simulink model "MCVT_Integration"  (R2020a)
settings: Solver=ode8  StartTime=0.0  StopTime=T_end  FixedStep=dt  RelTol=1e-3  EnableMultiTasking=on
values from neighbouring files (symbols this model uses):
  assumed_power_voltage = 1.25e2    (= 125)    [MCVT_Input_File.m]    (also in MCVT_Run_QuickCheck.m)
  battery_power_toggle = 1    [MCVT_Input_File.m]    (also in MCVT_Run_QuickCheck.m)
  battery_trickle_charge_param = 0.1    [MCVT_Input_File.m]    (also in MCVT_Run_QuickCheck.m)
  battery_trickle_leakage_param = 0.01    [MCVT_Input_File.m]    (also in MCVT_Run_QuickCheck.m)
  damage_case_id = 3    [MCVT_Input_File.m]    (also in MCVT_Run_QuickCheck.m = 1)
  dt = 1/fs    (= 0.001)    [MCVT_Run_QuickCheck.m]    (also in MCVT_Sim_Set.m)
  dt_2 = 1/fs_2    (= 0.001)    [MCVT_Run_QuickCheck.m]    (also in MCVT_Sim_Set.m)
  dt_3 = 1/fs_3    (= 0.001)    [MCVT_Run_QuickCheck.m]    (also in MCVT_Sim_Set.m)
  dt_5 = 1/fs_5    (= 0.005)    [MCVT_Run_QuickCheck.m]    (also in MCVT_Sim_Set.m)
  dt_8 = 1/fs_8    (= 0.05)    [MCVT_Run_QuickCheck.m]    (also in MCVT_Sim_Set.m)
  dt_9 = 1/fs_9    (= 0.001)    [MCVT_Run_QuickCheck.m]    (also in MCVT_Sim_Set.m)
  dust_met_duration = 3    [MCVT_Input_File.m]    (also in MCVT_Run_QuickCheck.m = 2)
  ECLSS_indoor_set_temperature = 298.15    [MCVT_Input_File.m]    (also in MCVT_Run_QuickCheck.m)
  energy_store_max_o = 150    [MCVT_Input_File.m]    (also in MCVT_Run_QuickCheck.m)
  energy_store_o = 120    [MCVT_Input_File.m]    (also in MCVT_Run_QuickCheck.m)
  fs = 1000    [MCVT_Run_QuickCheck.m]    (also in MCVT_Sim_Set.m)
  gain_charge_param = 1    [MCVT_Input_File.m]    (also in MCVT_Run_QuickCheck.m)
  ground_temperature = 373    [MCVT_Habitat_Design_Input_File.m]    (also in MCVT_Run_QuickCheck.m)
  I_max_charge = 800    [MCVT_Input_File.m]    (also in MCVT_Run_QuickCheck.m)
  I_max_discharge = 800    [MCVT_Input_File.m]    (also in MCVT_Run_QuickCheck.m)
  initial_pressure = 1e5    (= 100000)    [MCVT_Input_File.m]    (also in MCVT_Run_QuickCheck.m)
  initial_temperature = 295    [MCVT_Input_File.m]    (also in MCVT_Run_QuickCheck.m)
  interior_leak_rate = 0.00415    [MCVT_Input_File.m]    (also in MCVT_Run_QuickCheck.m)
  launch_dust_accum_start_time = 10    [MCVT_Input_File.m]    (also in MCVT_Run_QuickCheck.m = 2)
  launch_dust_accum_stop_time = 12    [MCVT_Input_File.m]    (also in MCVT_Run_QuickCheck.m = 3)
  meteorite_impact_time = 2    [MCVT_Input_File.m]    (also in MCVT_Run_QuickCheck.m = 1)
  nuclear_power_toggle = 1    [MCVT_Input_File.m]    (also in MCVT_Run_QuickCheck.m)
  print_interval = 30    [MCVT_Input_File.m]
  SOC_lower_thres = 0.5    [MCVT_Input_File.m]    (also in MCVT_Run_QuickCheck.m)
  SOC_upper_thres = 0.9    [MCVT_Input_File.m]    (also in MCVT_Run_QuickCheck.m)
  solar_power_toggle = 1    [MCVT_Input_File.m]    (also in MCVT_Run_QuickCheck.m)
  space_temperature = 4    [MCVT_Habitat_Design_Input_File.m]    (also in MCVT_Run_QuickCheck.m)
  Start_angle = 90    [MCVT_Input_File.m]    (also in MCVT_Run_QuickCheck.m)
undefined symbols (not defined in the file or in its folder): A_flow_restrictor, ACTIVITY_LOOKUP_TABLE, air, Aref, Bmap, Bmap2, Bmap3, comms, DiscIntNeverNeededParam, DmgSPL, dust_parameters, ECLSS_dust_damage_repair_time, ECLSS_heater_capacity, ECLSS_IPS_restrictor, ECLSS_IPS_setpointPressure, ECLSS_paint_damage_repair_time, ECLSS_radiatorLoop_discreteNumber, ECLSS_Vst, emptyActivity, expected_heat_add, FI, habitat, HIEM2SMM, HIEM2STM, HIEMDamageElements, impact_numbering, initial_SPLT_damage_index, input_air, input_Cond, input_CP, input_discretization, input_EV, input_Evap, input_radiatorLoop, K_ECLSS_dust_single, K_ECLSS_paint_single, K_Structure_damage_single, Kref, kW_to_W, meteoroidDustDep, mFRF, ndelays, nominalDustDep, noverlap, nuclear_toggle_gain, output_limits, panel_paint0, Ps0, reference_temperature, RepairTime_ECLSS_factor, Sensor_Location_SMM, SMM_T0, SMM_T1, SMM_T2, SMM_T3, SMM_T4, SMM_T4_0, SMM_T4_1, SMM_T4_2, solar_FDD_K ... (+73)
libraries: neural x350, simulink x39, xpcutilitieslib x7, xpcrtudplib x7, dspstat3 x1
(part 29 of 43)

== system MCVT_Integration/Sys2 - Structural System/Sys2_Structural_System/Fault Detection and Diagnosis (FDD) with Binary Classifer/Pattern Recognition Neural Network/Segment 4/Layer 2/Delays 1 ==
  "p{2}" : Inport  PortDimensions=20
      mask Display: e = 0.06;c1 = [120 230 180]/255;c2 = ([120 230 180]+0)/(256+128);c3 = ([120 230 180]+128)/(256+128);patch([0 1 1 0],[0 0 1 1],c1);patch([0 1 1 1-e 1-e 0+e],[0 0 1 1-e 0+e 0+e],c2);patch([0 0 1 1-e 0+e 0+e],[0 1 1 1-e 1-e 0+e],c3);plot([0 1 1 0 0],[0 0 1 1 0]);
  mux : Mux  Inputs=1
      mask Display: e = 0.06;c1 = [120 150 230]/255;c2 = ([120 150 230]+0)/383;c3 = ([120 150 230]+128)/383;patch([0 1 1 0],[0 0 1 1],c1);patch([0 1 1 1-e 1-e 0+e],[0 0 1 1-e 0+e 0+e],c2);patch([0 0 1 1-e 0+e 0+e],[0 1 1 1-e 1-e 0+e],c3);plot([0 1 1 0 0],[0 0 1 1 0]);disp('Mux')
  "pd{2,1}" : Outport
      mask Display: e = 0.06;c1 = [120 230 180]/255;c2 = ([120 230 180]+0)/(256+128);c3 = ([120 230 180]+128)/(256+128);patch([0 1 1 0],[0 0 1 1],c1);patch([0 1 1 1-e 1-e 0+e],[0 0 1 1-e 0+e 0+e],c2);patch([0 0 1 1-e 0+e 0+e],[0 1 1 1-e 1-e 0+e],c3);plot([0 1 1 0 0],[0 0 1 1 0]);
  wires:
  "p{2}"/1 -> mux/1
  mux/1 -> "pd{2,1}"/1

== system MCVT_Integration/Sys2 - Structural System/Sys2_Structural_System/Fault Detection and Diagnosis (FDD) with Binary Classifer/Pattern Recognition Neural Network/Segment 4/Layer 2/LW{2,1} ==
  "ad{2,1}" : Inport  PortDimensions=20
      mask Display: e = 0.06;c1 = [120 230 180]/255;c2 = ([120 230 180]+0)/(256+128);c3 = ([120 230 180]+128)/(256+128);patch([0 1 1 0],[0 0 1 1],c1);patch([0 1 1 1-e 1-e 0+e],[0 0 1 1-e 0+e 0+e],c2);patch([0 0 1 1-e 0+e 0+e],[0 1 1 1-e 1-e 0+e],c3);plot([0 1 1 0 0],[0 0 1 1 0]);
  "IW{2,1}(1,:)'" : Constant  Value=[0.306057142007762739144283159475889988243579864501953125;0.310512128739632020657523980844416655600070953369140625;-0.29480112676645597868940740227117203176021575927734375;0.2026576403739423770655747603086638264358043670654296875;0.0186213755057179040919290713418376981280744075775146484375;-0.62303529414836733568705540164955891668796539306640625;0.27996475650757235431598246577777899801731109619140625;-0.469892300787330519096229863862390629947185516357421875;0.349973395308618318022553239643457345664501190185546875;-0.476340885668002533837039891295717097818851470947265625;-0.02019900162674355403868986513771233148872852325439453125;0.472241782546273636267386564213666133582592010498046875;-0.07055543952916958072574260540932300500571727752685546875;-0.678306634812040787352316328906454145908355712890625;0.296746899937958996673614819883368909358978271484375;0.198279570842823271714649990826728753745555877685546875;-0.025708135562139890206712067310945712961256504058837890625;-0.338335032564995363824067453606403432786464691162109375;0.08612698156654709225055199794951477088034152984619140625;-0.68933092867270528980583321754238568246364593505859375]
      mask Display: e = 0.06;c1 = [120 150 230]/255;c2 = ([120 150 230]+0)/383;c3 = ([120 150 230]+128)/383;patch([0 1 1 0],[0 0 1 1],c1);patch([0 1 1 1-e 1-e 0+e],[0 0 1 1-e 0+e 0+e],c2);patch([0 0 1 1-e 0+e 0+e],[0 1 1 1-e 1-e 0+e],c3);plot([0 1 1 0 0],[0 0 1 1 0]);disp('weights');
  "IW{2,1}(10,:)'" : Constant  Value=[-0.454412246804836328362142694459180347621440887451171875;-0.4824565986072466561296323561691679060459136962890625;-0.275395655377960679732751714254845865070819854736328125;-0.0803078351074401342035713469158508814871311187744140625;-0.37013280180053442780518935251166112720966339111328125;-0.4570841826751632641645528565277345478534698486328125;-0.682178892744035092476906356750987470149993896484375;0.574565380760992727715574801550246775150299072265625;0.2066591468841111056686798974624252878129482269287109375;0.251641779457071612657870218754396773874759674072265625;0.474189808136070090061053861063555814325809478759765625;-0.3065595300955170632306590050575323402881622314453125;-0.03251040501423851925011376806651242077350616455078125;0.53208328271825788835514003949356265366077423095703125;-0.54559425954344564768661030029761604964733123779296875;-0.1546572822269978997322681379955611191689968109130859375;-0.2079459967763304939492030598557903431355953216552734375;-0.125847872776466196409472786399419419467449188232421875;-0.2171635029508142966658823524994659237563610076904296875;-0.008098184410637955521483632992385537363588809967041015625]
      mask Display: e = 0.06;c1 = [120 150 230]/255;c2 = ([120 150 230]+0)/383;c3 = ([120 150 230]+128)/383;patch([0 1 1 0],[0 0 1 1],c1);patch([0 1 1 1-e 1-e 0+e],[0 0 1 1-e 0+e 0+e],c2);patch([0 0 1 1-e 0+e 0+e],[0 1 1 1-e 1-e 0+e],c3);plot([0 1 1 0 0],[0 0 1 1 0]);disp('weights');
  "IW{2,1}(11,:)'" : Constant  Value=[-0.355743113415101619434466329039423726499080657958984375;-0.2977000196718904145853912268648855388164520263671875;-0.166305359141924735677520175158861093223094940185546875;0.63506621475033886436989405410713516175746917724609375;-0.12211245879492793608989131826092489063739776611328125;-0.35734830918081283979148565776995383203029632568359375;-0.007084321038460337539854894117752337479032576084136962890625;-0.51062960099843046624101816632901318371295928955078125;0.1967739673482086504296972861993708647787570953369140625;0.523644006460218580656373887904919683933258056640625;-0.12499041066855280657232896146524581126868724822998046875;0.469006153564551109269586959271691739559173583984375;-0.28864209698220744115815250552259385585784912109375;-0.70178566481582149716444973819307051599025726318359375;-0.461286454007475088534562246422865428030490875244140625;-0.1140809577348286529829834989868686534464359283447265625;-0.1564664967301874209937295745476149022579193115234375;0.1812230404505438541296058474472374655306339263916015625;0.32782724784233108206166207310161553323268890380859375;0.1198120416025670598170194125486887060105800628662109375]
      mask Display: e = 0.06;c1 = [120 150 230]/255;c2 = ([120 150 230]+0)/383;c3 = ([120 150 230]+128)/383;patch([0 1 1 0],[0 0 1 1],c1);patch([0 1 1 1-e 1-e 0+e],[0 0 1 1-e 0+e 0+e],c2);patch([0 0 1 1-e 0+e 0+e],[0 1 1 1-e 1-e 0+e],c3);plot([0 1 1 0 0],[0 0 1 1 0]);disp('weights');
  "IW{2,1}(12,:)'" : Constant  Value=[0.544592015893043512875237865955568850040435791015625;0.017266354118825787133939542172811343334615230560302734375;0.054713993936632322745428069765694090165197849273681640625;0.6186534402811296740054558540578000247478485107421875;-0.440406666837937998959517926778062246739864349365234375;-0.2128995960319074731703636871316120959818363189697265625;0.5122083357285378557577359970309771597385406494140625;0.452050976139218574356704039018950425088405609130859375;-0.320969153304328014275625946538639254868030548095703125;-0.0286717582855797979701772959515437833033502101898193359375;0.264975992191505582695754128508269786834716796875;0.1252840007930953458714640191828948445618152618408203125;0.30296273686784169765218166503473185002803802490234375;0.389740197399307375381027895855368115007877349853515625;0.59820597595326907569557306487695313990116119384765625;-0.383276695756931740088901960916700772941112518310546875;-0.2816220426152564737520833659800700843334197998046875;-0.125183420626807151432302589455503039062023162841796875;0.50451177141658665181722653869655914604663848876953125;-0.10778819385341818770651656222980818711221218109130859375]
      mask Display: e = 0.06;c1 = [120 150 230]/255;c2 = ([120 150 230]+0)/383;c3 = ([120 150 230]+128)/383;patch([0 1 1 0],[0 0 1 1],c1);patch([0 1 1 1-e 1-e 0+e],[0 0 1 1-e 0+e 0+e],c2);patch([0 0 1 1-e 0+e 0+e],[0 1 1 1-e 1-e 0+e],c3);plot([0 1 1 0 0],[0 0 1 1 0]);disp('weights');
  "IW{2,1}(13,:)'" : Constant  Value=[-0.36239375267019025006476340422523207962512969970703125;-0.50940676322083910942950524258776567876338958740234375;0.49917583333415238566743710180162452161312103271484375;-0.473264315167338411871611469905474223196506500244140625;-0.0681152986488545442167463761506951414048671722412109375;-0.341762868656012575030445077572949230670928955078125;0.10071269586357746439464477816727594472467899322509765625;-0.255670409891921079914567371815792284905910491943359375;-0.195414759332962317817106168149621225893497467041015625;0.273947243800715389827615808826521970331668853759765625;0.496574492529116950922940532109350897371768951416015625;0.463933083275532565092902359538129530847072601318359375;-0.5125281010838158568532207937096245586872100830078125;-0.209332357712803285298974742545397020876407623291015625;-0.425416539077372757304829065105877816677093505859375;0.14576288969835815567677173021365888416767120361328125;-0.36721855328845531829529136302880942821502685546875;-0.477309855799878224313914643062162213027477264404296875;0.000781326268361681557095277383950815419666469097137451171875;-0.33735050472358241169246184654184617102146148681640625]
      mask Display: e = 0.06;c1 = [120 150 230]/255;c2 = ([120 150 230]+0)/383;c3 = ([120 150 230]+128)/383;patch([0 1 1 0],[0 0 1 1],c1);patch([0 1 1 1-e 1-e 0+e],[0 0 1 1-e 0+e 0+e],c2);patch([0 0 1 1-e 0+e 0+e],[0 1 1 1-e 1-e 0+e],c3);plot([0 1 1 0 0],[0 0 1 1 0]);disp('weights');
  "IW{2,1}(14,:)'" : Constant  Value=[0.6664352993426387872233362941187806427478790283203125;-0.457753524342841278116367220718530006706714630126953125;0.52365648767706840516211741487495601177215576171875;0.11253739685686410532117207594637875445187091827392578125;-0.58929248151265511967977772656013257801532745361328125;0.074616389784726344647225459993933327496051788330078125;-0.21640729063620078864715878808056004345417022705078125;-0.040891488319182267563434152179979719221591949462890625;0.279256125095423357596047253537108190357685089111328125;0.01086720430103971755941838495118645369075238704681396484375;-0.260969654081474544682350824587047100067138671875;-0.43309678761145231629114960014703683555126190185546875;0.329919346090738141441534025943838059902191162109375;0.222617805712427563324951051981770433485507965087890625;0.14641681679105389424222494199057109653949737548828125;0.3079234911730275303654025265132077038288116455078125;-0.63760415074333021134833643372985534369945526123046875;0.358470231563331243052061836351640522480010986328125;-0.2888555698222463608004773050197400152683258056640625;0.227998343599048081120628239659708924591541290283203125]
      mask Display: e = 0.06;c1 = [120 150 230]/255;c2 = ([120 150 230]+0)/383;c3 = ([120 150 230]+128)/383;patch([0 1 1 0],[0 0 1 1],c1);patch([0 1 1 1-e 1-e 0+e],[0 0 1 1-e 0+e 0+e],c2);patch([0 0 1 1-e 0+e 0+e],[0 1 1 1-e 1-e 0+e],c3);plot([0 1 1 0 0],[0 0 1 1 0]);disp('weights');
  "IW{2,1}(15,:)'" : Constant  Value=[-0.307260772906464418863237142431898973882198333740234375;-0.61309323992605868625105358660221099853515625;-0.054875600330744227217660835549395415000617504119873046875;-0.373131406576250601592192879252252168953418731689453125;0.17024217048548229147542087957845069468021392822265625;-0.267314583188805909852447939556441269814968109130859375;0.5680484901431730460075186783797107636928558349609375;0.490789145080530120335282617816119454801082611083984375;-0.389900000418462566731392371366382576525211334228515625;0.531135855414851310030144304619170725345611572265625;0.306231871194271809688558505513356067240238189697265625;0.51068416873600586125547806659596972167491912841796875;-0.48518086271943217102631251691491343080997467041015625;-0.5393878541416281535703092231415212154388427734375;0.11958801347122692548818889690664946101605892181396484375;-0.349111884885122514265987092585419304668903350830078125;0.04236443264176380230079388411468244157731533050537109375;-0.248563630843463723163466738697024993598461151123046875;-0.00327480905387556205099475192810132284648716449737548828125;-0.47065772481461520815315680010826326906681060791015625]
      mask Display: e = 0.06;c1 = [120 150 230]/255;c2 = ([120 150 230]+0)/383;c3 = ([120 150 230]+128)/383;patch([0 1 1 0],[0 0 1 1],c1);patch([0 1 1 1-e 1-e 0+e],[0 0 1 1-e 0+e 0+e],c2);patch([0 0 1 1-e 0+e 0+e],[0 1 1 1-e 1-e 0+e],c3);plot([0 1 1 0 0],[0 0 1 1 0]);disp('weights');
  "IW{2,1}(16,:)'" : Constant  Value=[0.266308933582539764106655866271466948091983795166015625;-0.364733881565826478787784026280860416591167449951171875;0.2989971125247870009644657329772599041461944580078125;-0.233175761922731761277560735834413208067417144775390625;0.54430320536453657975783926303847692906856536865234375;-0.488756758150722336875304563363897614181041717529296875;0.442993403197067869836445197506691329181194305419921875;-0.51242787261809297216785807904670946300029754638671875;0.4217879051450135197143254117690958082675933837890625;-0.54522718317692986378375508138560689985752105712890625;0.418513030467745517082533979191794060170650482177734375;-0.496028536108564177542490369887673296034336090087890625;-0.1616779408732620104682808914731140248477458953857421875;0.3573027981917251683086078628548420965671539306640625;-0.336751366256044182279083543107844889163970947265625;0.25935866550005670649881039935280568897724151611328125;-0.314265171343225568900692223905934952199459075927734375;-0.319165344878365953018573009103420190513134002685546875;0.07779002724300769500143104551170836202800273895263671875;0.1269806052742208646311183883881312794983386993408203125]
      mask Display: e = 0.06;c1 = [120 150 230]/255;c2 = ([120 150 230]+0)/383;c3 = ([120 150 230]+128)/383;patch([0 1 1 0],[0 0 1 1],c1);patch([0 1 1 1-e 1-e 0+e],[0 0 1 1-e 0+e 0+e],c2);patch([0 0 1 1-e 0+e 0+e],[0 1 1 1-e 1-e 0+e],c3);plot([0 1 1 0 0],[0 0 1 1 0]);disp('weights');
  "IW{2,1}(17,:)'" : Constant  Value=[0.53082566544809439701424480517744086682796478271484375;-0.58284397025850243068134659552015364170074462890625;0.34375308624808298407771189886261709034442901611328125;-0.58269637950061559106273989527835510671138763427734375;-0.1311653113894331867950171499614953063428401947021484375;0.342431540873028683602541377695160917937755584716796875;0.25199078079994274848019131241017021238803863525390625;-0.382307105356534560058889837819151580333709716796875;-0.329743810721619901027423793493653647601604461669921875;0.39199771612980838231266034199506975710391998291015625;0.0235790953045496819162618606924297637306153774261474609375;-0.230803622605871117112741330856806598603725433349609375;0.5089936760389750514121942615020088851451873779296875;-0.583278797416965755218143385718576610088348388671875;0.25668236353005646765979008705471642315387725830078125;-0.1393060012447478179797855091237579472362995147705078125;-0.17183182406345454129592553726979531347751617431640625;0.1109685594165018829304614200736978091299533843994140625;-0.187448843294550415716770430663018487393856048583984375;-0.379710978863437909058831110087339766323566436767578125]
      mask Display: e = 0.06;c1 = [120 150 230]/255;c2 = ([120 150 230]+0)/383;c3 = ([120 150 230]+128)/383;patch([0 1 1 0],[0 0 1 1],c1);patch([0 1 1 1-e 1-e 0+e],[0 0 1 1-e 0+e 0+e],c2);patch([0 0 1 1-e 0+e 0+e],[0 1 1 1-e 1-e 0+e],c3);plot([0 1 1 0 0],[0 0 1 1 0]);disp('weights');
  "IW{2,1}(18,:)'" : Constant  Value=[-0.455619957350509086069223485537804663181304931640625;-0.61081561261032835119522133027203381061553955078125;0.39972462020356835932233252606238238513469696044921875;0.256034880153321486861983657945529557764530181884765625;0.1829835871873809194365634311907342635095119476318359375;-0.05988612027758366540552970036515034735202789306640625;0.2211006989277527756687646842692629434168338775634765625;0.395513403572112398620674866833724081516265869140625;0.350100931568261886095427826148807071149349212646484375;0.374723226508432871728615509709925390779972076416015625;0.1805421696730828140520230817855917848646640777587890625;0.1341574735849028476852851099465624429285526275634765625;0.410021219004975956412550885943346656858921051025390625;-0.27239455166578740996641272431588731706142425537109375;-0.339507089293388519823935212116339243948459625244140625;0.11792329099394795177691008802867145277559757232666015625;0.69772844306720538387622809750610031187534332275390625;0.315636637811678166798401434789411723613739013671875;-0.421998804985463105143850270906114019453525543212890625;-0.39195674802171154293972676896373741328716278076171875]
      mask Display: e = 0.06;c1 = [120 150 230]/255;c2 = ([120 150 230]+0)/383;c3 = ([120 150 230]+128)/383;patch([0 1 1 0],[0 0 1 1],c1);patch([0 1 1 1-e 1-e 0+e],[0 0 1 1-e 0+e 0+e],c2);patch([0 0 1 1-e 0+e 0+e],[0 1 1 1-e 1-e 0+e],c3);plot([0 1 1 0 0],[0 0 1 1 0]);disp('weights');
  "IW{2,1}(19,:)'" : Constant  Value=[-0.163349644939564442314150483070989139378070831298828125;0.121243105185530775091962141232215799391269683837890625;-0.29496038714175620842894431916647590696811676025390625;-0.3640133515071457548373246027040295302867889404296875;-0.44100678682741334402095390032627619802951812744140625;-0.01094860937553309744585394724936122656799852848052978515625;-0.566270280313223128842992082354612648487091064453125;0.5929723664355692758221039184718392789363861083984375;0.37717930793271603473471031975350342690944671630859375;0.38624202033408405743131197596085257828235626220703125;0.053139585963738834861747051263591856695711612701416015625;-0.428028549078483699918962201991234906017780303955078125;-0.432512026401700666600191880206693895161151885986328125;-0.252740907967358097163668162465910427272319793701171875;0.50987974359008425562223010274465195834636688232421875;-0.256751156368664579243699108701548539102077484130859375;-0.14565396931513363565358076812117360532283782958984375;-0.281686261770320023334335246545379050076007843017578125;-0.101609049067601209248579152699676342308521270751953125;0.57849152722496255396578135332674719393253326416015625]
      mask Display: e = 0.06;c1 = [120 150 230]/255;c2 = ([120 150 230]+0)/383;c3 = ([120 150 230]+128)/383;patch([0 1 1 0],[0 0 1 1],c1);patch([0 1 1 1-e 1-e 0+e],[0 0 1 1-e 0+e 0+e],c2);patch([0 0 1 1-e 0+e 0+e],[0 1 1 1-e 1-e 0+e],c3);plot([0 1 1 0 0],[0 0 1 1 0]);disp('weights');
  "IW{2,1}(2,:)'" : Constant  Value=[-0.159190858356428599496013021052931435406208038330078125;0.138287225234651478533720592167810536921024322509765625;-0.7412804610270684602113533401279710233211517333984375;0.466639037632644082531641060995752923190593719482421875;-0.0104381557840903284317679577952731051482260227203369140625;0.08968104810817767447161941163358278572559356689453125;-0.318172440514310983505907870494411326944828033447265625;0.283506599383576751716162789307418279349803924560546875;0.6157233951232081725635225666337646543979644775390625;-0.2286641519230156738284875928002293221652507781982421875;-0.402988905513761042076481544427224434912204742431640625;0.02162496561790484828069480727208429016172885894775390625;0.01116868922299207866899450891651213169097900390625;-0.31021934578641474633542429728549905121326446533203125;0.61231479790218734660101063127513043582439422607421875;-0.36921380470495057668500749059603549540042877197265625;-0.16435845422759631784259681808180175721645355224609375;-0.31707279842367463995600473936065100133419036865234375;0.55124586169898692755708680124371312558650970458984375;0.094910146614590240599085291250958107411861419677734375]
      mask Display: e = 0.06;c1 = [120 150 230]/255;c2 = ([120 150 230]+0)/383;c3 = ([120 150 230]+128)/383;patch([0 1 1 0],[0 0 1 1],c1);patch([0 1 1 1-e 1-e 0+e],[0 0 1 1-e 0+e 0+e],c2);patch([0 0 1 1-e 0+e 0+e],[0 1 1 1-e 1-e 0+e],c3);plot([0 1 1 0 0],[0 0 1 1 0]);disp('weights');
  "IW{2,1}(20,:)'" : Constant  Value=[-0.425843471005478979574121467521763406693935394287109375;-0.5966351198699706426253897006972692906856536865234375;-0.27562888186791079103699075858457945287227630615234375;-0.28610829686546612737885197930154390633106231689453125;-0.1054210676776156929701500075680087320506572723388671875;-0.0228439142759308023988840119500309810973703861236572265625;-0.30801254896618368572802637572749517858028411865234375;-0.1263951542205036526755890236017876304686069488525390625;-0.7028606256047538192177626115153543651103973388671875;-0.340742771807667377270689712531748227775096893310546875;-0.1428542252600741024881614293917664326727390289306640625;-0.27127021062786005334288574886159040033817291259765625;-0.50662588654624907125167965205037035048007965087890625;-0.1598808946230525218634710427068057470023632049560546875;-0.0586698739952324632707103546636062674224376678466796875;-0.4954462661548399982081036796444095671176910400390625;0.41687845632897901904101445325068198144435882568359375;0.31867345334111207844074442618875764310359954833984375;0.2253999313182314112591342336600064300000667572021484375;-0.50866271865239587324225567499524913728237152099609375]
      mask Display: e = 0.06;c1 = [120 150 230]/255;c2 = ([120 150 230]+0)/383;c3 = ([120 150 230]+128)/383;patch([0 1 1 0],[0 0 1 1],c1);patch([0 1 1 1-e 1-e 0+e],[0 0 1 1-e 0+e 0+e],c2);patch([0 0 1 1-e 0+e 0+e],[0 1 1 1-e 1-e 0+e],c3);plot([0 1 1 0 0],[0 0 1 1 0]);disp('weights');
  "IW{2,1}(3,:)'" : Constant  Value=[0.021728797129401962451300533984976937063038349151611328125;0.458633958670552510650253452695324085652828216552734375;-0.05318393793418750570278774603139027021825313568115234375;-0.489301203282900110114184144549653865396976470947265625;0.485517217465149197863638619310222566127777099609375;-0.276889504077018788752440059397486038506031036376953125;0.21596870115905630882480181753635406494140625;0.016946886754216239356907180990674532949924468994140625;-0.455619597921827101938418991267099045217037200927734375;-0.226081028922258286950608408005791716277599334716796875;0.46635278208896291385343602087232284247875213623046875;0.290360413347985735210698976516141556203365325927734375;-0.453452173198495678274611009328509680926799774169921875;0.00703559875327504878617101979898507124744355678558349609375;0.46335204225870152416888458901667036116123199462890625;-0.5247876104940016883659836821607314050197601318359375;0.460198458165902379857215009906212799251079559326171875;-0.01220026829110053105675870455115727963857352733612060546875;-0.349403392812220492391617199245956726372241973876953125;-0.4287321811898776591220894260914064943790435791015625]
      mask Display: e = 0.06;c1 = [120 150 230]/255;c2 = ([120 150 230]+0)/383;c3 = ([120 150 230]+128)/383;patch([0 1 1 0],[0 0 1 1],c1);patch([0 1 1 1-e 1-e 0+e],[0 0 1 1-e 0+e 0+e],c2);patch([0 0 1 1-e 0+e 0+e],[0 1 1 1-e 1-e 0+e],c3);plot([0 1 1 0 0],[0 0 1 1 0]);disp('weights');
  "IW{2,1}(4,:)'" : Constant  Value=[0.3020888621613340152549653794267214834690093994140625;-0.390998365216094156959769634340773336589336395263671875;0.0294171549674970521370820364381870604120194911956787109375;0.52855285881612312781641094261431135237216949462890625;-0.2211219189329124701348661119482130743563175201416015625;0.55994043442587582148917135782539844512939453125;-0.667884182564916439872604314587078988552093505859375;-0.42631403377280541189264795320923440158367156982421875;0.216644315526017428030769451652304269373416900634765625;-0.142698339805427398818693518478539772331714630126953125;-0.52746818647277582403631868146476335823535919189453125;-0.03017150263048573588520184785011224448680877685546875;0.62876125880153832525820689625106751918792724609375;0.2075770587739970973739644932720693759620189666748046875;0.0929065392864592276556123806585674174129962921142578125;0.490626823131944556077854713294073008000850677490234375;-0.34614707339849803968689911926048807799816131591796875;0.38948819813905555253796819670242257416248321533203125;-0.3344611592311104164565449536894448101520538330078125;-0.1576382385081467052412307339182007126510143280029296875]
      mask Display: e = 0.06;c1 = [120 150 230]/255;c2 = ([120 150 230]+0)/383;c3 = ([120 150 230]+128)/383;patch([0 1 1 0],[0 0 1 1],c1);patch([0 1 1 1-e 1-e 0+e],[0 0 1 1-e 0+e 0+e],c2);patch([0 0 1 1-e 0+e 0+e],[0 1 1 1-e 1-e 0+e],c3);plot([0 1 1 0 0],[0 0 1 1 0]);disp('weights');
  "IW{2,1}(5,:)'" : Constant  Value=[-0.07033250450795879016308020936776301823556423187255859375;0.1149394782230703793146631142008118331432342529296875;0.0617400908887539845437686381046660244464874267578125;0.490259729448167702603456064025522209703922271728515625;0.58198223303013552065721114558982662856578826904296875;0.4793342193892742297833819975494407117366790771484375;0.59141685330090354444365630115498788654804229736328125;0.29234167301284319506748943240381777286529541015625;-0.071306230296348582786691849833005107939243316650390625;0.3292006548754089489960961145698092877864837646484375;0.48736478646974024098170730212586931884288787841796875;0.50683414871738718954219393708626739680767059326171875;-0.09226312378716459983163389324545278213918209075927734375;0.20971891636035178141383994443458504974842071533203125;-0.1481811820230655996066815305312047712504863739013671875;-0.45315265486786326931678559049032628536224365234375;-0.335260674183771423262356847772025503218173980712890625;-0.5108503944537055030394867571885697543621063232421875;0.358957861039638392863793114884174428880214691162109375;-0.34866796914738851231874150471412576735019683837890625]
      mask Display: e = 0.06;c1 = [120 150 230]/255;c2 = ([120 150 230]+0)/383;c3 = ([120 150 230]+128)/383;patch([0 1 1 0],[0 0 1 1],c1);patch([0 1 1 1-e 1-e 0+e],[0 0 1 1-e 0+e 0+e],c2);patch([0 0 1 1-e 0+e 0+e],[0 1 1 1-e 1-e 0+e],c3);plot([0 1 1 0 0],[0 0 1 1 0]);disp('weights');
  "IW{2,1}(6,:)'" : Constant  Value=[-0.345978995440224534885231832959107123315334320068359375;0.0892463660759024446367249083778006024658679962158203125;0.03168208696493236831148720966666587628424167633056640625;-0.42305129289704901385249513623421080410480499267578125;0.33642924445719646353580856157350353896617889404296875;0.53704305640171379199188095299177803099155426025390625;0.51011986920863383598856444223201833665370941162109375;-0.391488263119827262226380071297171525657176971435546875;-0.5326338185622183640788307457114569842815399169921875;0.368601750312640585871548637442174367606639862060546875;0.48912631435939124546763423495576716959476470947265625;-0.13751543295665646926551062279031611979007720947265625;0.44857006333210758253215999502572230994701385498046875;0.308459014727003044686881594316218979656696319580078125;-0.378642575934863945263941786834038794040679931640625;0.32301348938158280788002230110578238964080810546875;0.46518729928909596793573655304498970508575439453125;-0.2445123518208422719322214788917335681617259979248046875;0.0809114079555009191313530436673318035900592803955078125;-0.0049149379712071505910131463679135777056217193603515625]
      mask Display: e = 0.06;c1 = [120 150 230]/255;c2 = ([120 150 230]+0)/383;c3 = ([120 150 230]+128)/383;patch([0 1 1 0],[0 0 1 1],c1);patch([0 1 1 1-e 1-e 0+e],[0 0 1 1-e 0+e 0+e],c2);patch([0 0 1 1-e 0+e 0+e],[0 1 1 1-e 1-e 0+e],c3);plot([0 1 1 0 0],[0 0 1 1 0]);disp('weights');
  "IW{2,1}(7,:)'" : Constant  Value=[-0.397286146474948254070369557666708715260028839111328125;-0.43614400863508417938163574945065192878246307373046875;-0.1613120777449850440365963777367142029106616973876953125;-0.345359329080283161683695425381301902234554290771484375;-0.25948535908394931848164333132444880902767181396484375;0.52528149992678174751148389987065456807613372802734375;-0.377789089137232581006031750803231261670589447021484375;-0.27581689840720768902571080616326071321964263916015625;-0.2004782856999751394777575796979363076388835906982421875;-0.50485919905651488459596976099419407546520233154296875;-0.554220830068306469229355570860207080841064453125;0.00323440531958108130894391507581531186588108539581298828125;0.09109518491461419953392208981313160620629787445068359375;0.5244495449693749034025813671178184449672698974609375;0.0220969657888940955448031644436923670582473278045654296875;0.38293034302528161649803450927720405161380767822265625;0.4429690652572688680521650894661433994770050048828125;0.41926386790190506292930194831569679081439971923828125;0.3944882781758856094000975645030848681926727294921875;0.43750692681324865329628437393694184720516204833984375]
      mask Display: e = 0.06;c1 = [120 150 230]/255;c2 = ([120 150 230]+0)/383;c3 = ([120 150 230]+128)/383;patch([0 1 1 0],[0 0 1 1],c1);patch([0 1 1 1-e 1-e 0+e],[0 0 1 1-e 0+e 0+e],c2);patch([0 0 1 1-e 0+e 0+e],[0 1 1 1-e 1-e 0+e],c3);plot([0 1 1 0 0],[0 0 1 1 0]);disp('weights');
  "IW{2,1}(8,:)'" : Constant  Value=[-0.04962403085949386449815534660956473089754581451416015625;-0.28081775498237993016203972729272209107875823974609375;0.212659328407800274352013047973741777241230010986328125;0.496557614420993331805220805108547210693359375;-0.5389800393161141567333061175304464995861053466796875;-0.420717582122753730455855247782892547547817230224609375;0.32513253633136052211938249456579796969890594482421875;-0.56538959638970620602549388422630727291107177734375;0.37656299886752397920730572877801023423671722412109375;-0.2085692127990641797641302446209010668098926544189453125;0.53056256677287050305125148952356539666652679443359375;0.2256311148381764664083704019503784365952014923095703125;0.308542020127856975175717479942250065505504608154296875;-0.38089116872857164963761533726938068866729736328125;0.038488572709682096484318236662147683091461658477783203125;0.423232702924032089253358890346135012805461883544921875;-0.592209689853139931159375919378362596035003662109375;-0.14900161187568505827272247188375331461429595947265625;-0.1573180447283173688521884514557314105331897735595703125;0.196429830882492328925081892521120607852935791015625]
      mask Display: e = 0.06;c1 = [120 150 230]/255;c2 = ([120 150 230]+0)/383;c3 = ([120 150 230]+128)/383;patch([0 1 1 0],[0 0 1 1],c1);patch([0 1 1 1-e 1-e 0+e],[0 0 1 1-e 0+e 0+e],c2);patch([0 0 1 1-e 0+e 0+e],[0 1 1 1-e 1-e 0+e],c3);plot([0 1 1 0 0],[0 0 1 1 0]);disp('weights');
  "IW{2,1}(9,:)'" : Constant  Value=[0.08161848332739808642433132490623393096029758453369140625;0.0305114918968062143189712287494330666959285736083984375;0.45490686170960803469398570086923427879810333251953125;-0.51287488453557006362615311445551924407482147216796875;-0.475618867301149828019646292887046001851558685302734375;0.430238717718641250353783789250883273780345916748046875;0.191450181161164689758180657008779235184192657470703125;0.388964624120451019084754307186813093721866607666015625;-0.10473962770201415695137114880708395503461360931396484375;0.001272180832418833007491087982998578809201717376708984375;0.16080603973133078543611418353975750505924224853515625;0.63762329018861485518954168583150021731853485107421875;-0.5415638896880683450518745303270407021045684814453125;0.339144450411268782996643267324543558061122894287109375;0.318341851003351383742057123527047224342823028564453125;-0.0006185602689792422947956396228619269095361232757568359375;-0.52142307102542251318055832598474808037281036376953125;0.302570422194620325395675308755016885697841644287109375;-0.461419795842032354205031197125208564102649688720703125;-0.040741678281950181872428373708316939882934093475341796875]
      mask Display: e = 0.06;c1 = [120 150 230]/255;c2 = ([120 150 230]+0)/383;c3 = ([120 150 230]+128)/383;patch([0 1 1 0],[0 0 1 1],c1);patch([0 1 1 1-e 1-e 0+e],[0 0 1 1-e 0+e 0+e],c2);patch([0 0 1 1-e 0+e 0+e],[0 1 1 1-e 1-e 0+e],c3);plot([0 1 1 0 0],[0 0 1 1 0]);disp('weights');
  Mux : Mux  Inputs=20
      mask Display: e = 0.06;c1 = [120 150 230]/255;c2 = ([120 150 230]+0)/383;c3 = ([120 150 230]+128)/383;patch([0 1 1 0],[0 0 1 1],c1);patch([0 1 1 1-e 1-e 0+e],[0 0 1 1-e 0+e 0+e],c2);patch([0 0 1 1-e 0+e 0+e],[0 1 1 1-e 1-e 0+e],c3);plot([0 1 1 0 0],[0 0 1 1 0]);disp('Mux');
  dotprod{1..20} (20 alike) : neural/Weight Functions/dotprod
  "lz{2,1}" : Outport
      mask Display: e = 0.06;c1 = [120 230 180]/255;c2 = ([120 230 180]+0)/(256+128);c3 = ([120 230 180]+128)/(256+128);patch([0 1 1 0],[0 0 1 1],c1);patch([0 1 1 1-e 1-e 0+e],[0 0 1 1-e 0+e 0+e],c2);patch([0 0 1 1-e 0+e 0+e],[0 1 1 1-e 1-e 0+e],c3);plot([0 1 1 0 0],[0 0 1 1 0]);
  wires:
  "IW{2,1}(1,:)'"/1 -> dotprod1/1
  "ad{2,1}"/1 -> dotprod1/2, dotprod2/2, dotprod3/2, dotprod4/2, dotprod5/2, dotprod6/2, dotprod7/2, dotprod8/2, dotprod9/2, dotprod10/2, dotprod11/2, dotprod12/2, dotprod13/2, dotprod14/2, dotprod15/2, dotprod16/2, dotprod17/2, dotprod18/2, dotprod19/2, dotprod20/2
  dotprod1/1 -> Mux/1
  "IW{2,1}(2,:)'"/1 -> dotprod2/1
  dotprod2/1 -> Mux/2
  "IW{2,1}(3,:)'"/1 -> dotprod3/1
  dotprod3/1 -> Mux/3
  "IW{2,1}(4,:)'"/1 -> dotprod4/1
  dotprod4/1 -> Mux/4
  "IW{2,1}(5,:)'"/1 -> dotprod5/1
  dotprod5/1 -> Mux/5
  "IW{2,1}(6,:)'"/1 -> dotprod6/1
  dotprod6/1 -> Mux/6
  "IW{2,1}(7,:)'"/1 -> dotprod7/1
  dotprod7/1 -> Mux/7
  "IW{2,1}(8,:)'"/1 -> dotprod8/1
  dotprod8/1 -> Mux/8
  "IW{2,1}(9,:)'"/1 -> dotprod9/1
  dotprod9/1 -> Mux/9
  "IW{2,1}(10,:)'"/1 -> dotprod10/1
  dotprod10/1 -> Mux/10
  "IW{2,1}(11,:)'"/1 -> dotprod11/1
  dotprod11/1 -> Mux/11
  "IW{2,1}(12,:)'"/1 -> dotprod12/1
  dotprod12/1 -> Mux/12
  "IW{2,1}(13,:)'"/1 -> dotprod13/1
  dotprod13/1 -> Mux/13
  "IW{2,1}(14,:)'"/1 -> dotprod14/1
  dotprod14/1 -> Mux/14
  "IW{2,1}(15,:)'"/1 -> dotprod15/1
  dotprod15/1 -> Mux/15
  "IW{2,1}(16,:)'"/1 -> dotprod16/1
  dotprod16/1 -> Mux/16
  "IW{2,1}(17,:)'"/1 -> dotprod17/1
  dotprod17/1 -> Mux/17
  "IW{2,1}(18,:)'"/1 -> dotprod18/1
  dotprod18/1 -> Mux/18
  "IW{2,1}(19,:)'"/1 -> dotprod19/1
  dotprod19/1 -> Mux/19
  "IW{2,1}(20,:)'"/1 -> dotprod20/1
  dotprod20/1 -> Mux/20
  Mux/1 -> "lz{2,1}"/1

== system MCVT_Integration/Sys2 - Structural System/Sys2_Structural_System/Fault Detection and Diagnosis (FDD) with Binary Classifer/Pattern Recognition Neural Network/Segment 4/Layer 3/Delays 1 ==
  "p{3}" : Inport  PortDimensions=20
      mask Display: e = 0.06;c1 = [120 230 180]/255;c2 = ([120 230 180]+0)/(256+128);c3 = ([120 230 180]+128)/(256+128);patch([0 1 1 0],[0 0 1 1],c1);patch([0 1 1 1-e 1-e 0+e],[0 0 1 1-e 0+e 0+e],c2);patch([0 0 1 1-e 0+e 0+e],[0 1 1 1-e 1-e 0+e],c3);plot([0 1 1 0 0],[0 0 1 1 0]);
  mux : Mux  Inputs=1
      mask Display: e = 0.06;c1 = [120 150 230]/255;c2 = ([120 150 230]+0)/383;c3 = ([120 150 230]+128)/383;patch([0 1 1 0],[0 0 1 1],c1);patch([0 1 1 1-e 1-e 0+e],[0 0 1 1-e 0+e 0+e],c2);patch([0 0 1 1-e 0+e 0+e],[0 1 1 1-e 1-e 0+e],c3);plot([0 1 1 0 0],[0 0 1 1 0]);disp('Mux')
  "pd{3,2}" : Outport
      mask Display: e = 0.06;c1 = [120 230 180]/255;c2 = ([120 230 180]+0)/(256+128);c3 = ([120 230 180]+128)/(256+128);patch([0 1 1 0],[0 0 1 1],c1);patch([0 1 1 1-e 1-e 0+e],[0 0 1 1-e 0+e 0+e],c2);patch([0 0 1 1-e 0+e 0+e],[0 1 1 1-e 1-e 0+e],c3);plot([0 1 1 0 0],[0 0 1 1 0]);
  wires:
  "p{3}"/1 -> mux/1
  mux/1 -> "pd{3,2}"/1

== system MCVT_Integration/Sys2 - Structural System/Sys2_Structural_System/Fault Detection and Diagnosis (FDD) with Binary Classifer/Pattern Recognition Neural Network/Segment 4/Layer 3/LW{3,2} ==
  "ad{3,2}" : Inport  PortDimensions=20
      mask Display: e = 0.06;c1 = [120 230 180]/255;c2 = ([120 230 180]+0)/(256+128);c3 = ([120 230 180]+128)/(256+128);patch([0 1 1 0],[0 0 1 1],c1);patch([0 1 1 1-e 1-e 0+e],[0 0 1 1-e 0+e 0+e],c2);patch([0 0 1 1-e 0+e 0+e],[0 1 1 1-e 1-e 0+e],c3);plot([0 1 1 0 0],[0 0 1 1 0]);
  "IW{3,2}(1,:)'" : Constant  Value=[0.64203411075592276002765856901532970368862152099609375;0.41363558611404205311856685511884279549121856689453125;-0.450595772889152479390162397976382635533809661865234375;-1.1366566134297817480813819202012382447719573974609375;0.12323004034856656352392434428111300803720951080322265625;-0.73094091013321904615196444865432567894458770751953125;-0.55681593449733879364060840089223347604274749755859375;-0.0453288904100472012093092644136049784719944000244140625;0.63592594326142648508692900577443651854991912841796875;-0.7965119421725048720617223807494156062602996826171875;-0.3128533752841813342371324324631132185459136962890625;0.7249632835882893200363241703598760068416595458984375;0.307909902189266782013277179430588148534297943115234375;-0.549941545612202986603733734227716922760009765625;1.1638493837727004187598822682048194110393524169921875;0.79728932514492190097854518171516247093677520751953125;0.1297234904122661902636792774501373060047626495361328125;-0.69521959722643977830358608116512186825275421142578125;0.0465345112665491722481192482518963515758514404296875;0.76710751935013277158503797181765548884868621826171875]
      mask Display: e = 0.06;c1 = [120 150 230]/255;c2 = ([120 150 230]+0)/383;c3 = ([120 150 230]+128)/383;patch([0 1 1 0],[0 0 1 1],c1);patch([0 1 1 1-e 1-e 0+e],[0 0 1 1-e 0+e 0+e],c2);patch([0 0 1 1-e 0+e 0+e],[0 1 1 1-e 1-e 0+e],c3);plot([0 1 1 0 0],[0 0 1 1 0]);disp('weights');
  "IW{3,2}(2,:)'" : Constant  Value=[-0.17496447262181391568702792937983758747577667236328125;0.59756616827413588932671473230584524571895599365234375;-0.215156372562907538537047003046609461307525634765625;0.256549401295889933916072322972468100488185882568359375;-0.72681402747691803423180090248933993279933929443359375;-0.97383757501332646189240449530188925564289093017578125;0.2461141887916838688621368191888905130326747894287109375;0.2157423306256315009843405050560249947011470794677734375;-0.54917846987314467011032093068934045732021331787109375;0.08366963312444854194982468698071897961199283599853515625;0.79153034706550140509051516346517018973827362060546875;-0.07208539395854030029653358724317513406276702880859375;0.140894407532480780975703282820177264511585235595703125;-0.72169548055297827460208281991071999073028564453125;-0.481223296780931952820736796638811938464641571044921875;-0.51625362388467543883763255507801659405231475830078125;0.2365201319126627177436006377320154570043087005615234375;-0.2831345564578200413308195493300445377826690673828125;0.1078035057421497799889920088389771990478038787841796875;-0.85453334532752356356155587491230107843875885009765625]
      mask Display: e = 0.06;c1 = [120 150 230]/255;c2 = ([120 150 230]+0)/383;c3 = ([120 150 230]+128)/383;patch([0 1 1 0],[0 0 1 1],c1);patch([0 1 1 1-e 1-e 0+e],[0 0 1 1-e 0+e 0+e],c2);patch([0 0 1 1-e 0+e 0+e],[0 1 1 1-e 1-e 0+e],c3);plot([0 1 1 0 0],[0 0 1 1 0]);disp('weights');
  Mux : Mux  Inputs=2
      mask Display: e = 0.06;c1 = [120 150 230]/255;c2 = ([120 150 230]+0)/383;c3 = ([120 150 230]+128)/383;patch([0 1 1 0],[0 0 1 1],c1);patch([0 1 1 1-e 1-e 0+e],[0 0 1 1-e 0+e 0+e],c2);patch([0 0 1 1-e 0+e 0+e],[0 1 1 1-e 1-e 0+e],c3);plot([0 1 1 0 0],[0 0 1 1 0]);disp('Mux');
  dotprod1 : neural/Weight Functions/dotprod
  dotprod2 : neural/Weight Functions/dotprod
  "lz{3,2}" : Outport
      mask Display: e = 0.06;c1 = [120 230 180]/255;c2 = ([120 230 180]+0)/(256+128);c3 = ([120 230 180]+128)/(256+128);patch([0 1 1 0],[0 0 1 1],c1);patch([0 1 1 1-e 1-e 0+e],[0 0 1 1-e 0+e 0+e],c2);patch([0 0 1 1-e 0+e 0+e],[0 1 1 1-e 1-e 0+e],c3);plot([0 1 1 0 0],[0 0 1 1 0]);
  wires:
  "IW{3,2}(1,:)'"/1 -> dotprod1/1
  "ad{3,2}"/1 -> dotprod1/2, dotprod2/2
  dotprod1/1 -> Mux/1
  "IW{3,2}(2,:)'"/1 -> dotprod2/1
  dotprod2/1 -> Mux/2
  Mux/1 -> "lz{3,2}"/1

== system MCVT_Integration/Sys2 - Structural System/Sys2_Structural_System/Fault Detection and Diagnosis (FDD) with Binary Classifer/Pattern Recognition Neural Network/Segment 5/Layer 1/Delays 1 ==
  "p{1}" : Inport  PortDimensions=2304
      mask Display: e = 0.06;c1 = [120 230 180]/255;c2 = ([120 230 180]+0)/(256+128);c3 = ([120 230 180]+128)/(256+128);patch([0 1 1 0],[0 0 1 1],c1);patch([0 1 1 1-e 1-e 0+e],[0 0 1 1-e 0+e 0+e],c2);patch([0 0 1 1-e 0+e 0+e],[0 1 1 1-e 1-e 0+e],c3);plot([0 1 1 0 0],[0 0 1 1 0]);
  mux : Mux  Inputs=1
      mask Display: e = 0.06;c1 = [120 150 230]/255;c2 = ([120 150 230]+0)/383;c3 = ([120 150 230]+128)/383;patch([0 1 1 0],[0 0 1 1],c1);patch([0 1 1 1-e 1-e 0+e],[0 0 1 1-e 0+e 0+e],c2);patch([0 0 1 1-e 0+e 0+e],[0 1 1 1-e 1-e 0+e],c3);plot([0 1 1 0 0],[0 0 1 1 0]);disp('Mux')
  "pd{1,1}" : Outport
      mask Display: e = 0.06;c1 = [120 230 180]/255;c2 = ([120 230 180]+0)/(256+128);c3 = ([120 230 180]+128)/(256+128);patch([0 1 1 0],[0 0 1 1],c1);patch([0 1 1 1-e 1-e 0+e],[0 0 1 1-e 0+e 0+e],c2);patch([0 0 1 1-e 0+e 0+e],[0 1 1 1-e 1-e 0+e],c3);plot([0 1 1 0 0],[0 0 1 1 0]);
  wires:
  "p{1}"/1 -> mux/1
  mux/1 -> "pd{1,1}"/1
